simulink model "Simulatore_COMAU_UBHAND_IV_manipolazione_interna"  (Simulink 7.6)
settings: Solver=ode45  StartTime=0.0  StopTime=inf  RelTol=1e-3
values from neighbouring files (symbols this model uses):
  a_f = zeros(4,5)    [KinematicData.m]
  alpha_f = zeros(4,5)    [KinematicData.m]
  d_f = zeros(4,5)    [KinematicData.m]
  dc4_1 = 660    [KinematicData.m]
  T6HAND = [1 0 0 0;0 1 0 0; 0 0 1 357.48+95; 0 0 0 1]    [KinematicData.m]
  teta_d = atan(647.07/130)    [KinematicData.m]
  teta_f = zeros(4,5)    [KinematicData.m]
undefined symbols (not defined in the file or in its folder): a_c, a_w, alpha_c, alpha_w, d_c, d_w, Dt_fingers, teta_c
libraries: simulink x281, dspmtrx3 x171, aerolibutil x163, aerolibconvert2 x64, simulink_extras x46, vrlib x26, aerolibtransform2 x7, dspnetwork x1
(part 7 of 15)

== system Simulatore_COMAU_UBHAND_IV_manipolazione_interna/SCOPES/Direct_kinematic2/Direct_kinematic_hand/Direct_kinematic_index/Subsystem ==
  q : Inport
  alpha : Inport  Port=2
  a : Inport  Port=3
  A_01 : aerolibutil/Create 3x3 Matrix
  Constant : Constant  Value=0
  Constant1 : Constant  Value=[0 0 0 1]  VectorParams1D=off
  Constant5 : Constant  Value=0
  Display : Display
  Gain : Gain  Gain=-1
  Gain1 : Gain  Gain=-1
  Matrix Concatenation : Concatenate  Mode=Multidimensional array  ConcatenateDimension=2
  Matrix Concatenation1 : Concatenate  Mode=Multidimensional array
  Product{,1..5} (6 alike) : Product  RndMeth=Zero
  Trigonometric Function{,1,6..8,11..12} (7 alike) : Trigonometry  Operator=cos
  Trigonometric Function{2..5,9..10,13} (7 alike) : Trigonometry
  Vector Concatenate : Concatenate  NumInputs=3  Mode=Multidimensional array
  Out1 : Outport
  wires:
  Matrix Concatenation1/1 -> Display/1, Out1/1
  Constant1/1 -> Matrix Concatenation1/2
  Matrix Concatenation/1 -> Matrix Concatenation1/1
  Vector Concatenate/1 -> Matrix Concatenation/2
  A_01/1 -> Matrix Concatenation/1
  Constant5/1 -> Vector Concatenate/3
  Product5/1 -> Vector Concatenate/2
  Product4/1 -> Vector Concatenate/1
  Trigonometric Function13/1 -> Product5/2
  Trigonometric Function12/1 -> Product4/2
  a/1 -> Product5/1, Product4/1
  Trigonometric Function11/1 -> A_01/9
  Trigonometric Function10/1 -> A_01/8
  Constant/1 -> A_01/7
  Gain1/1 -> A_01/6
  Product2/1 -> A_01/5
  Trigonometric Function5/1 -> A_01/4
  Product1/1 -> A_01/3
  Gain/1 -> A_01/2
  Trigonometric Function/1 -> A_01/1
  Product/1 -> Gain/1
  Product3/1 -> Gain1/1
  Trigonometric Function9/1 -> Product3/2
  Trigonometric Function8/1 -> Product3/1
  Trigonometric Function7/1 -> Product2/2
  Trigonometric Function6/1 -> Product2/1
  Trigonometric Function4/1 -> Product1/2
  Trigonometric Function3/1 -> Product1/1
  alpha/1 -> Trigonometric Function11/1, Trigonometric Function10/1, Trigonometric Function9/1, Trigonometric Function7/1, Trigonometric Function4/1, Trigonometric Function1/1
  q/1 -> Trigonometric Function13/1, Trigonometric Function12/1, Trigonometric Function8/1, Trigonometric Function6/1, Trigonometric Function5/1, Trigonometric Function3/1, Trigonometric Function2/1, Trigonometric Function/1
  Trigonometric Function1/1 -> Product/2
  Trigonometric Function2/1 -> Product/1

== system Simulatore_COMAU_UBHAND_IV_manipolazione_interna/SCOPES/Direct_kinematic2/Direct_kinematic_hand/Direct_kinematic_index/Subsystem1 ==
  q : Inport
  alpha : Inport  Port=2
  a : Inport  Port=3
  A_01 : aerolibutil/Create 3x3 Matrix
  Constant : Constant  Value=0
  Constant1 : Constant  Value=[0 0 0 1]  VectorParams1D=off
  Constant5 : Constant  Value=0
  Display : Display
  Gain : Gain  Gain=-1
  Gain1 : Gain  Gain=-1
  Matrix Concatenation : Concatenate  Mode=Multidimensional array  ConcatenateDimension=2
  Matrix Concatenation1 : Concatenate  Mode=Multidimensional array
  Product{,1..5} (6 alike) : Product  RndMeth=Zero
  Trigonometric Function{,1,6..8,11..12} (7 alike) : Trigonometry  Operator=cos
  Trigonometric Function{2..5,9..10,13} (7 alike) : Trigonometry
  Vector Concatenate : Concatenate  NumInputs=3  Mode=Multidimensional array
  Out1 : Outport
  wires:
  Trigonometric Function2/1 -> Product/1
  Trigonometric Function1/1 -> Product/2
  q/1 -> Trigonometric Function/1, Trigonometric Function2/1, Trigonometric Function3/1, Trigonometric Function5/1, Trigonometric Function6/1, Trigonometric Function8/1, Trigonometric Function12/1, Trigonometric Function13/1
  alpha/1 -> Trigonometric Function1/1, Trigonometric Function4/1, Trigonometric Function7/1, Trigonometric Function9/1, Trigonometric Function10/1, Trigonometric Function11/1
  Trigonometric Function3/1 -> Product1/1
  Trigonometric Function4/1 -> Product1/2
  Trigonometric Function6/1 -> Product2/1
  Trigonometric Function7/1 -> Product2/2
  Trigonometric Function8/1 -> Product3/1
  Trigonometric Function9/1 -> Product3/2
  Product3/1 -> Gain1/1
  Product/1 -> Gain/1
  Trigonometric Function/1 -> A_01/1
  Gain/1 -> A_01/2
  Product1/1 -> A_01/3
  Trigonometric Function5/1 -> A_01/4
  Product2/1 -> A_01/5
  Gain1/1 -> A_01/6
  Constant/1 -> A_01/7
  Trigonometric Function10/1 -> A_01/8
  Trigonometric Function11/1 -> A_01/9
  a/1 -> Product5/1, Product4/1
  Trigonometric Function12/1 -> Product4/2
  Trigonometric Function13/1 -> Product5/2
  Product4/1 -> Vector Concatenate/1
  Product5/1 -> Vector Concatenate/2
  Constant5/1 -> Vector Concatenate/3
  A_01/1 -> Matrix Concatenation/1
  Vector Concatenate/1 -> Matrix Concatenation/2
  Matrix Concatenation/1 -> Matrix Concatenation1/1
  Constant1/1 -> Matrix Concatenation1/2
  Matrix Concatenation1/1 -> Out1/1, Display/1

== system Simulatore_COMAU_UBHAND_IV_manipolazione_interna/SCOPES/Direct_kinematic2/Direct_kinematic_hand/Direct_kinematic_index/Subsystem2 ==
  q : Inport
  alpha : Inport  Port=2
  a : Inport  Port=3
  A_01 : aerolibutil/Create 3x3 Matrix
  Constant : Constant  Value=0
  Constant1 : Constant  Value=[0 0 0 1]  VectorParams1D=off
  Constant5 : Constant  Value=0
  Display : Display
  Gain : Gain  Gain=-1
  Gain1 : Gain  Gain=-1
  Matrix Concatenation : Concatenate  Mode=Multidimensional array  ConcatenateDimension=2
  Matrix Concatenation1 : Concatenate  Mode=Multidimensional array
  Product{,1..5} (6 alike) : Product  RndMeth=Zero
  Trigonometric Function{,1,6..8,11..12} (7 alike) : Trigonometry  Operator=cos
  Trigonometric Function{2..5,9..10,13} (7 alike) : Trigonometry
  Vector Concatenate : Concatenate  NumInputs=3  Mode=Multidimensional array
  Out1 : Outport
  wires:
  Matrix Concatenation1/1 -> Display/1, Out1/1
  Constant1/1 -> Matrix Concatenation1/2
  Matrix Concatenation/1 -> Matrix Concatenation1/1
  Vector Concatenate/1 -> Matrix Concatenation/2
  A_01/1 -> Matrix Concatenation/1
  Constant5/1 -> Vector Concatenate/3
  Product5/1 -> Vector Concatenate/2
  Product4/1 -> Vector Concatenate/1
  Trigonometric Function13/1 -> Product5/2
  Trigonometric Function12/1 -> Product4/2
  a/1 -> Product5/1, Product4/1
  Trigonometric Function11/1 -> A_01/9
  Trigonometric Function10/1 -> A_01/8
  Constant/1 -> A_01/7
  Gain1/1 -> A_01/6
  Product2/1 -> A_01/5
  Trigonometric Function5/1 -> A_01/4
  Product1/1 -> A_01/3
  Gain/1 -> A_01/2
  Trigonometric Function/1 -> A_01/1
  Product/1 -> Gain/1
  Product3/1 -> Gain1/1
  Trigonometric Function9/1 -> Product3/2
  Trigonometric Function8/1 -> Product3/1
  Trigonometric Function7/1 -> Product2/2
  Trigonometric Function6/1 -> Product2/1
  Trigonometric Function4/1 -> Product1/2
  Trigonometric Function3/1 -> Product1/1
  alpha/1 -> Trigonometric Function11/1, Trigonometric Function10/1, Trigonometric Function9/1, Trigonometric Function7/1, Trigonometric Function4/1, Trigonometric Function1/1
  q/1 -> Trigonometric Function13/1, Trigonometric Function12/1, Trigonometric Function8/1, Trigonometric Function6/1, Trigonometric Function5/1, Trigonometric Function3/1, Trigonometric Function2/1, Trigonometric Function/1
  Trigonometric Function1/1 -> Product/2
  Trigonometric Function2/1 -> Product/1

== system Simulatore_COMAU_UBHAND_IV_manipolazione_interna/SCOPES/Direct_kinematic2/Direct_kinematic_hand/Direct_kinematic_index/Subsystem3 ==
  q : Inport
  alpha : Inport  Port=2
  a : Inport  Port=3
  A_01 : aerolibutil/Create 3x3 Matrix
  Constant : Constant  Value=0
  Constant1 : Constant  Value=[0 0 0 1]  VectorParams1D=off
  Constant5 : Constant  Value=0
  Display : Display
  Gain : Gain  Gain=-1
  Gain1 : Gain  Gain=-1
  Matrix Concatenation : Concatenate  Mode=Multidimensional array  ConcatenateDimension=2
  Matrix Concatenation1 : Concatenate  Mode=Multidimensional array
  Product{,1..5} (6 alike) : Product  RndMeth=Zero
  Trigonometric Function{,1,6..8,11..12} (7 alike) : Trigonometry  Operator=cos
  Trigonometric Function{2..5,9..10,13} (7 alike) : Trigonometry
  Vector Concatenate : Concatenate  NumInputs=3  Mode=Multidimensional array
  Out1 : Outport
  wires:
  Trigonometric Function2/1 -> Product/1
  Trigonometric Function1/1 -> Product/2
  q/1 -> Trigonometric Function/1, Trigonometric Function2/1, Trigonometric Function3/1, Trigonometric Function5/1, Trigonometric Function6/1, Trigonometric Function8/1, Trigonometric Function12/1, Trigonometric Function13/1
  alpha/1 -> Trigonometric Function1/1, Trigonometric Function4/1, Trigonometric Function7/1, Trigonometric Function9/1, Trigonometric Function10/1, Trigonometric Function11/1
  Trigonometric Function3/1 -> Product1/1
  Trigonometric Function4/1 -> Product1/2
  Trigonometric Function6/1 -> Product2/1
  Trigonometric Function7/1 -> Product2/2
  Trigonometric Function8/1 -> Product3/1
  Trigonometric Function9/1 -> Product3/2
  Product3/1 -> Gain1/1
  Product/1 -> Gain/1
  Trigonometric Function/1 -> A_01/1
  Gain/1 -> A_01/2
  Product1/1 -> A_01/3
  Trigonometric Function5/1 -> A_01/4
  Product2/1 -> A_01/5
  Gain1/1 -> A_01/6
  Constant/1 -> A_01/7
  Trigonometric Function10/1 -> A_01/8
  Trigonometric Function11/1 -> A_01/9
  a/1 -> Product5/1, Product4/1
  Trigonometric Function12/1 -> Product4/2
  Trigonometric Function13/1 -> Product5/2
  Product4/1 -> Vector Concatenate/1
  Product5/1 -> Vector Concatenate/2
  Constant5/1 -> Vector Concatenate/3
  A_01/1 -> Matrix Concatenation/1
  Vector Concatenate/1 -> Matrix Concatenation/2
  Matrix Concatenation/1 -> Matrix Concatenation1/1
  Constant1/1 -> Matrix Concatenation1/2
  Matrix Concatenation1/1 -> Out1/1, Display/1

== system Simulatore_COMAU_UBHAND_IV_manipolazione_interna/SCOPES/Direct_kinematic2/Direct_kinematic_hand/Direct_kinematic_little/Subsystem ==
  q : Inport
  alpha : Inport  Port=2
  a : Inport  Port=3
  A_01 : aerolibutil/Create 3x3 Matrix
  Constant : Constant  Value=0
  Constant1 : Constant  Value=[0 0 0 1]  VectorParams1D=off
  Constant5 : Constant  Value=0
  Display : Display
  Gain : Gain  Gain=-1
  Gain1 : Gain  Gain=-1
  Matrix Concatenation : Concatenate  Mode=Multidimensional array  ConcatenateDimension=2
  Matrix Concatenation1 : Concatenate  Mode=Multidimensional array
  Product{,1..5} (6 alike) : Product  RndMeth=Zero
  Trigonometric Function{,1,6..8,11..12} (7 alike) : Trigonometry  Operator=cos
  Trigonometric Function{2..5,9..10,13} (7 alike) : Trigonometry
  Vector Concatenate : Concatenate  NumInputs=3  Mode=Multidimensional array
  Out1 : Outport
  wires:
  Matrix Concatenation1/1 -> Display/1, Out1/1
  Constant1/1 -> Matrix Concatenation1/2
  Matrix Concatenation/1 -> Matrix Concatenation1/1
  Vector Concatenate/1 -> Matrix Concatenation/2
  A_01/1 -> Matrix Concatenation/1
  Constant5/1 -> Vector Concatenate/3
  Product5/1 -> Vector Concatenate/2
  Product4/1 -> Vector Concatenate/1
  Trigonometric Function13/1 -> Product5/2
  Trigonometric Function12/1 -> Product4/2
  a/1 -> Product5/1, Product4/1
  Trigonometric Function11/1 -> A_01/9
  Trigonometric Function10/1 -> A_01/8
  Constant/1 -> A_01/7
  Gain1/1 -> A_01/6
  Product2/1 -> A_01/5
  Trigonometric Function5/1 -> A_01/4
  Product1/1 -> A_01/3
  Gain/1 -> A_01/2
  Trigonometric Function/1 -> A_01/1
  Product/1 -> Gain/1
  Product3/1 -> Gain1/1
  Trigonometric Function9/1 -> Product3/2
  Trigonometric Function8/1 -> Product3/1
  Trigonometric Function7/1 -> Product2/2
  Trigonometric Function6/1 -> Product2/1
  Trigonometric Function4/1 -> Product1/2
  Trigonometric Function3/1 -> Product1/1
  alpha/1 -> Trigonometric Function11/1, Trigonometric Function10/1, Trigonometric Function9/1, Trigonometric Function7/1, Trigonometric Function4/1, Trigonometric Function1/1
  q/1 -> Trigonometric Function13/1, Trigonometric Function12/1, Trigonometric Function8/1, Trigonometric Function6/1, Trigonometric Function5/1, Trigonometric Function3/1, Trigonometric Function2/1, Trigonometric Function/1
  Trigonometric Function1/1 -> Product/2
  Trigonometric Function2/1 -> Product/1

== system Simulatore_COMAU_UBHAND_IV_manipolazione_interna/SCOPES/Direct_kinematic2/Direct_kinematic_hand/Direct_kinematic_little/Subsystem1 ==
  q : Inport
  alpha : Inport  Port=2
  a : Inport  Port=3
  A_01 : aerolibutil/Create 3x3 Matrix
  Constant : Constant  Value=0
  Constant1 : Constant  Value=[0 0 0 1]  VectorParams1D=off
  Constant5 : Constant  Value=0
  Display : Display
  Gain : Gain  Gain=-1
  Gain1 : Gain  Gain=-1
  Matrix Concatenation : Concatenate  Mode=Multidimensional array  ConcatenateDimension=2
  Matrix Concatenation1 : Concatenate  Mode=Multidimensional array
  Product{,1..5} (6 alike) : Product  RndMeth=Zero
  Trigonometric Function{,1,6..8,11..12} (7 alike) : Trigonometry  Operator=cos
  Trigonometric Function{2..5,9..10,13} (7 alike) : Trigonometry
  Vector Concatenate : Concatenate  NumInputs=3  Mode=Multidimensional array
  Out1 : Outport
  wires:
  Trigonometric Function2/1 -> Product/1
  Trigonometric Function1/1 -> Product/2
  q/1 -> Trigonometric Function/1, Trigonometric Function2/1, Trigonometric Function3/1, Trigonometric Function5/1, Trigonometric Function6/1, Trigonometric Function8/1, Trigonometric Function12/1, Trigonometric Function13/1
  alpha/1 -> Trigonometric Function1/1, Trigonometric Function4/1, Trigonometric Function7/1, Trigonometric Function9/1, Trigonometric Function10/1, Trigonometric Function11/1
  Trigonometric Function3/1 -> Product1/1
  Trigonometric Function4/1 -> Product1/2
  Trigonometric Function6/1 -> Product2/1
  Trigonometric Function7/1 -> Product2/2
  Trigonometric Function8/1 -> Product3/1
  Trigonometric Function9/1 -> Product3/2
  Product3/1 -> Gain1/1
  Product/1 -> Gain/1
  Trigonometric Function/1 -> A_01/1
  Gain/1 -> A_01/2
  Product1/1 -> A_01/3
  Trigonometric Function5/1 -> A_01/4
  Product2/1 -> A_01/5
  Gain1/1 -> A_01/6
  Constant/1 -> A_01/7
  Trigonometric Function10/1 -> A_01/8
  Trigonometric Function11/1 -> A_01/9
  a/1 -> Product5/1, Product4/1
  Trigonometric Function12/1 -> Product4/2
  Trigonometric Function13/1 -> Product5/2
  Product4/1 -> Vector Concatenate/1
  Product5/1 -> Vector Concatenate/2
  Constant5/1 -> Vector Concatenate/3
  A_01/1 -> Matrix Concatenation/1
  Vector Concatenate/1 -> Matrix Concatenation/2
  Matrix Concatenation/1 -> Matrix Concatenation1/1
  Constant1/1 -> Matrix Concatenation1/2
  Matrix Concatenation1/1 -> Out1/1, Display/1

== system Simulatore_COMAU_UBHAND_IV_manipolazione_interna/SCOPES/Direct_kinematic2/Direct_kinematic_hand/Direct_kinematic_little/Subsystem2 ==
  q : Inport
  alpha : Inport  Port=2
  a : Inport  Port=3
  A_01 : aerolibutil/Create 3x3 Matrix
  Constant : Constant  Value=0
  Constant1 : Constant  Value=[0 0 0 1]  VectorParams1D=off
  Constant5 : Constant  Value=0
  Display : Display
  Gain : Gain  Gain=-1
  Gain1 : Gain  Gain=-1
  Matrix Concatenation : Concatenate  Mode=Multidimensional array  ConcatenateDimension=2
  Matrix Concatenation1 : Concatenate  Mode=Multidimensional array
  Product{,1..5} (6 alike) : Product  RndMeth=Zero
  Trigonometric Function{,1,6..8,11..12} (7 alike) : Trigonometry  Operator=cos
  Trigonometric Function{2..5,9..10,13} (7 alike) : Trigonometry
  Vector Concatenate : Concatenate  NumInputs=3  Mode=Multidimensional array
  Out1 : Outport
  wires:
  Matrix Concatenation1/1 -> Display/1, Out1/1
  Constant1/1 -> Matrix Concatenation1/2
  Matrix Concatenation/1 -> Matrix Concatenation1/1
  Vector Concatenate/1 -> Matrix Concatenation/2
  A_01/1 -> Matrix Concatenation/1
  Constant5/1 -> Vector Concatenate/3
  Product5/1 -> Vector Concatenate/2
  Product4/1 -> Vector Concatenate/1
  Trigonometric Function13/1 -> Product5/2
  Trigonometric Function12/1 -> Product4/2
  a/1 -> Product5/1, Product4/1
  Trigonometric Function11/1 -> A_01/9
  Trigonometric Function10/1 -> A_01/8
  Constant/1 -> A_01/7
  Gain1/1 -> A_01/6
  Product2/1 -> A_01/5
  Trigonometric Function5/1 -> A_01/4
  Product1/1 -> A_01/3
  Gain/1 -> A_01/2
  Trigonometric Function/1 -> A_01/1
  Product/1 -> Gain/1
  Product3/1 -> Gain1/1
  Trigonometric Function9/1 -> Product3/2
  Trigonometric Function8/1 -> Product3/1
  Trigonometric Function7/1 -> Product2/2
  Trigonometric Function6/1 -> Product2/1
  Trigonometric Function4/1 -> Product1/2
  Trigonometric Function3/1 -> Product1/1
  alpha/1 -> Trigonometric Function11/1, Trigonometric Function10/1, Trigonometric Function9/1, Trigonometric Function7/1, Trigonometric Function4/1, Trigonometric Function1/1
  q/1 -> Trigonometric Function13/1, Trigonometric Function12/1, Trigonometric Function8/1, Trigonometric Function6/1, Trigonometric Function5/1, Trigonometric Function3/1, Trigonometric Function2/1, Trigonometric Function/1
  Trigonometric Function1/1 -> Product/2
  Trigonometric Function2/1 -> Product/1

== system Simulatore_COMAU_UBHAND_IV_manipolazione_interna/SCOPES/Direct_kinematic2/Direct_kinematic_hand/Direct_kinematic_little/Subsystem3 ==
  q : Inport
  alpha : Inport  Port=2
  a : Inport  Port=3
  A_01 : aerolibutil/Create 3x3 Matrix
  Constant : Constant  Value=0
  Constant1 : Constant  Value=[0 0 0 1]  VectorParams1D=off
  Constant5 : Constant  Value=0
  Display : Display
  Gain : Gain  Gain=-1
  Gain1 : Gain  Gain=-1
  Matrix Concatenation : Concatenate  Mode=Multidimensional array  ConcatenateDimension=2
  Matrix Concatenation1 : Concatenate  Mode=Multidimensional array
  Product{,1..5} (6 alike) : Product  RndMeth=Zero
  Trigonometric Function{,1,6..8,11..12} (7 alike) : Trigonometry  Operator=cos
  Trigonometric Function{2..5,9..10,13} (7 alike) : Trigonometry
  Vector Concatenate : Concatenate  NumInputs=3  Mode=Multidimensional array
  Out1 : Outport
  wires:
  Trigonometric Function2/1 -> Product/1
  Trigonometric Function1/1 -> Product/2
  q/1 -> Trigonometric Function/1, Trigonometric Function2/1, Trigonometric Function3/1, Trigonometric Function5/1, Trigonometric Function6/1, Trigonometric Function8/1, Trigonometric Function12/1, Trigonometric Function13/1
  alpha/1 -> Trigonometric Function1/1, Trigonometric Function4/1, Trigonometric Function7/1, Trigonometric Function9/1, Trigonometric Function10/1, Trigonometric Function11/1
  Trigonometric Function3/1 -> Product1/1
  Trigonometric Function4/1 -> Product1/2
  Trigonometric Function6/1 -> Product2/1
  Trigonometric Function7/1 -> Product2/2
  Trigonometric Function8/1 -> Product3/1
  Trigonometric Function9/1 -> Product3/2
  Product3/1 -> Gain1/1
  Product/1 -> Gain/1
  Trigonometric Function/1 -> A_01/1
  Gain/1 -> A_01/2
  Product1/1 -> A_01/3
  Trigonometric Function5/1 -> A_01/4
  Product2/1 -> A_01/5
  Gain1/1 -> A_01/6
  Constant/1 -> A_01/7
  Trigonometric Function10/1 -> A_01/8
  Trigonometric Function11/1 -> A_01/9
  a/1 -> Product5/1, Product4/1
  Trigonometric Function12/1 -> Product4/2
  Trigonometric Function13/1 -> Product5/2
  Product4/1 -> Vector Concatenate/1
  Product5/1 -> Vector Concatenate/2
  Constant5/1 -> Vector Concatenate/3
  A_01/1 -> Matrix Concatenation/1
  Vector Concatenate/1 -> Matrix Concatenation/2
  Matrix Concatenation/1 -> Matrix Concatenation1/1
  Constant1/1 -> Matrix Concatenation1/2
  Matrix Concatenation1/1 -> Out1/1, Display/1

== system Simulatore_COMAU_UBHAND_IV_manipolazione_interna/SCOPES/Direct_kinematic2/Direct_kinematic_hand/Direct_kinematic_middle/Subsystem ==
  q : Inport
  alpha : Inport  Port=2
  a : Inport  Port=3
  A_01 : aerolibutil/Create 3x3 Matrix
  Constant : Constant  Value=0
  Constant1 : Constant  Value=[0 0 0 1]  VectorParams1D=off
  Constant5 : Constant  Value=0
  Display : Display
  Gain : Gain  Gain=-1
  Gain1 : Gain  Gain=-1
  Matrix Concatenation : Concatenate  Mode=Multidimensional array  ConcatenateDimension=2
  Matrix Concatenation1 : Concatenate  Mode=Multidimensional array
  Product{,1..5} (6 alike) : Product  RndMeth=Zero
  Trigonometric Function{,1,6..8,11..12} (7 alike) : Trigonometry  Operator=cos
  Trigonometric Function{2..5,9..10,13} (7 alike) : Trigonometry
  Vector Concatenate : Concatenate  NumInputs=3  Mode=Multidimensional array
  Out1 : Outport
  wires:
  Trigonometric Function2/1 -> Product/1
  Trigonometric Function1/1 -> Product/2
  q/1 -> Trigonometric Function/1, Trigonometric Function2/1, Trigonometric Function3/1, Trigonometric Function5/1, Trigonometric Function6/1, Trigonometric Function8/1, Trigonometric Function12/1, Trigonometric Function13/1
  alpha/1 -> Trigonometric Function1/1, Trigonometric Function4/1, Trigonometric Function7/1, Trigonometric Function9/1, Trigonometric Function10/1, Trigonometric Function11/1
  Trigonometric Function3/1 -> Product1/1
  Trigonometric Function4/1 -> Product1/2
  Trigonometric Function6/1 -> Product2/1
  Trigonometric Function7/1 -> Product2/2
  Trigonometric Function8/1 -> Product3/1
  Trigonometric Function9/1 -> Product3/2
  Product3/1 -> Gain1/1
  Product/1 -> Gain/1
  Trigonometric Function/1 -> A_01/1
  Gain/1 -> A_01/2
  Product1/1 -> A_01/3
  Trigonometric Function5/1 -> A_01/4
  Product2/1 -> A_01/5
  Gain1/1 -> A_01/6
  Constant/1 -> A_01/7
  Trigonometric Function10/1 -> A_01/8
  Trigonometric Function11/1 -> A_01/9
  a/1 -> Product4/1, Product5/1
  Trigonometric Function12/1 -> Product4/2
  Trigonometric Function13/1 -> Product5/2
  Product4/1 -> Vector Concatenate/1
  Product5/1 -> Vector Concatenate/2
  Constant5/1 -> Vector Concatenate/3
  A_01/1 -> Matrix Concatenation/1
  Vector Concatenate/1 -> Matrix Concatenation/2
  Matrix Concatenation/1 -> Matrix Concatenation1/1
  Constant1/1 -> Matrix Concatenation1/2
  Matrix Concatenation1/1 -> Out1/1, Display/1

== system Simulatore_COMAU_UBHAND_IV_manipolazione_interna/SCOPES/Direct_kinematic2/Direct_kinematic_hand/Direct_kinematic_middle/Subsystem1 ==
  q : Inport
  alpha : Inport  Port=2
  a : Inport  Port=3
  A_01 : aerolibutil/Create 3x3 Matrix
  Constant : Constant  Value=0
  Constant1 : Constant  Value=[0 0 0 1]  VectorParams1D=off
  Constant5 : Constant  Value=0
  Display : Display
  Gain : Gain  Gain=-1
  Gain1 : Gain  Gain=-1
  Matrix Concatenation : Concatenate  Mode=Multidimensional array  ConcatenateDimension=2
  Matrix Concatenation1 : Concatenate  Mode=Multidimensional array
  Product{,1..5} (6 alike) : Product  RndMeth=Zero
  Trigonometric Function{,1,6..8,11..12} (7 alike) : Trigonometry  Operator=cos
  Trigonometric Function{2..5,9..10,13} (7 alike) : Trigonometry
  Vector Concatenate : Concatenate  NumInputs=3  Mode=Multidimensional array
  Out1 : Outport
  wires:
  Matrix Concatenation1/1 -> Display/1, Out1/1
  Constant1/1 -> Matrix Concatenation1/2
  Matrix Concatenation/1 -> Matrix Concatenation1/1
  Vector Concatenate/1 -> Matrix Concatenation/2
  A_01/1 -> Matrix Concatenation/1
  Constant5/1 -> Vector Concatenate/3
  Product5/1 -> Vector Concatenate/2
  Product4/1 -> Vector Concatenate/1
  Trigonometric Function13/1 -> Product5/2
  Trigonometric Function12/1 -> Product4/2
  a/1 -> Product4/1, Product5/1
  Trigonometric Function11/1 -> A_01/9
  Trigonometric Function10/1 -> A_01/8
  Constant/1 -> A_01/7
  Gain1/1 -> A_01/6
  Product2/1 -> A_01/5
  Trigonometric Function5/1 -> A_01/4
  Product1/1 -> A_01/3
  Gain/1 -> A_01/2
  Trigonometric Function/1 -> A_01/1
  Product/1 -> Gain/1
  Product3/1 -> Gain1/1
  Trigonometric Function9/1 -> Product3/2
  Trigonometric Function8/1 -> Product3/1
  Trigonometric Function7/1 -> Product2/2
  Trigonometric Function6/1 -> Product2/1
  Trigonometric Function4/1 -> Product1/2
  Trigonometric Function3/1 -> Product1/1
  alpha/1 -> Trigonometric Function11/1, Trigonometric Function10/1, Trigonometric Function9/1, Trigonometric Function7/1, Trigonometric Function4/1, Trigonometric Function1/1
  q/1 -> Trigonometric Function13/1, Trigonometric Function12/1, Trigonometric Function8/1, Trigonometric Function6/1, Trigonometric Function5/1, Trigonometric Function3/1, Trigonometric Function2/1, Trigonometric Function/1
  Trigonometric Function1/1 -> Product/2
  Trigonometric Function2/1 -> Product/1

== system Simulatore_COMAU_UBHAND_IV_manipolazione_interna/SCOPES/Direct_kinematic2/Direct_kinematic_hand/Direct_kinematic_middle/Subsystem2 ==
  q : Inport
  alpha : Inport  Port=2
  a : Inport  Port=3
  A_01 : aerolibutil/Create 3x3 Matrix
  Constant : Constant  Value=0
  Constant1 : Constant  Value=[0 0 0 1]  VectorParams1D=off
  Constant5 : Constant  Value=0
  Display : Display
  Gain : Gain  Gain=-1
  Gain1 : Gain  Gain=-1
  Matrix Concatenation : Concatenate  Mode=Multidimensional array  ConcatenateDimension=2
  Matrix Concatenation1 : Concatenate  Mode=Multidimensional array
  Product{,1..5} (6 alike) : Product  RndMeth=Zero
  Trigonometric Function{,1,6..8,11..12} (7 alike) : Trigonometry  Operator=cos
  Trigonometric Function{2..5,9..10,13} (7 alike) : Trigonometry
  Vector Concatenate : Concatenate  NumInputs=3  Mode=Multidimensional array
  Out1 : Outport
  wires:
  Trigonometric Function2/1 -> Product/1
  Trigonometric Function1/1 -> Product/2
  q/1 -> Trigonometric Function/1, Trigonometric Function2/1, Trigonometric Function3/1, Trigonometric Function5/1, Trigonometric Function6/1, Trigonometric Function8/1, Trigonometric Function12/1, Trigonometric Function13/1
  alpha/1 -> Trigonometric Function1/1, Trigonometric Function4/1, Trigonometric Function7/1, Trigonometric Function9/1, Trigonometric Function10/1, Trigonometric Function11/1
  Trigonometric Function3/1 -> Product1/1
  Trigonometric Function4/1 -> Product1/2
  Trigonometric Function6/1 -> Product2/1
  Trigonometric Function7/1 -> Product2/2
  Trigonometric Function8/1 -> Product3/1
  Trigonometric Function9/1 -> Product3/2
  Product3/1 -> Gain1/1
  Product/1 -> Gain/1
  Trigonometric Function/1 -> A_01/1
  Gain/1 -> A_01/2
  Product1/1 -> A_01/3
  Trigonometric Function5/1 -> A_01/4
  Product2/1 -> A_01/5
  Gain1/1 -> A_01/6
  Constant/1 -> A_01/7
  Trigonometric Function10/1 -> A_01/8
  Trigonometric Function11/1 -> A_01/9
  a/1 -> Product4/1, Product5/1
  Trigonometric Function12/1 -> Product4/2
  Trigonometric Function13/1 -> Product5/2
  Product4/1 -> Vector Concatenate/1
  Product5/1 -> Vector Concatenate/2
  Constant5/1 -> Vector Concatenate/3
  A_01/1 -> Matrix Concatenation/1
  Vector Concatenate/1 -> Matrix Concatenation/2
  Matrix Concatenation/1 -> Matrix Concatenation1/1
  Constant1/1 -> Matrix Concatenation1/2
  Matrix Concatenation1/1 -> Out1/1, Display/1

== system Simulatore_COMAU_UBHAND_IV_manipolazione_interna/SCOPES/Direct_kinematic2/Direct_kinematic_hand/Direct_kinematic_middle/Subsystem3 ==
  q : Inport
  alpha : Inport  Port=2
  a : Inport  Port=3
  A_01 : aerolibutil/Create 3x3 Matrix
  Constant : Constant  Value=0
  Constant1 : Constant  Value=[0 0 0 1]  VectorParams1D=off
  Constant5 : Constant  Value=0
  Display : Display
  Gain : Gain  Gain=-1
  Gain1 : Gain  Gain=-1
  Matrix Concatenation : Concatenate  Mode=Multidimensional array  ConcatenateDimension=2
  Matrix Concatenation1 : Concatenate  Mode=Multidimensional array
  Product{,1..5} (6 alike) : Product  RndMeth=Zero
  Trigonometric Function{,1,6..8,11..12} (7 alike) : Trigonometry  Operator=cos
  Trigonometric Function{2..5,9..10,13} (7 alike) : Trigonometry
  Vector Concatenate : Concatenate  NumInputs=3  Mode=Multidimensional array
  Out1 : Outport
  wires:
  Matrix Concatenation1/1 -> Display/1, Out1/1
  Constant1/1 -> Matrix Concatenation1/2
  Matrix Concatenation/1 -> Matrix Concatenation1/1
  Vector Concatenate/1 -> Matrix Concatenation/2
  A_01/1 -> Matrix Concatenation/1
  Constant5/1 -> Vector Concatenate/3
  Product5/1 -> Vector Concatenate/2
  Product4/1 -> Vector Concatenate/1
  Trigonometric Function13/1 -> Product5/2
  Trigonometric Function12/1 -> Product4/2
  a/1 -> Product4/1, Product5/1
  Trigonometric Function11/1 -> A_01/9
  Trigonometric Function10/1 -> A_01/8
  Constant/1 -> A_01/7
  Gain1/1 -> A_01/6
  Product2/1 -> A_01/5
  Trigonometric Function5/1 -> A_01/4
  Product1/1 -> A_01/3
  Gain/1 -> A_01/2
  Trigonometric Function/1 -> A_01/1
  Product/1 -> Gain/1
  Product3/1 -> Gain1/1
  Trigonometric Function9/1 -> Product3/2
  Trigonometric Function8/1 -> Product3/1
  Trigonometric Function7/1 -> Product2/2
  Trigonometric Function6/1 -> Product2/1
  Trigonometric Function4/1 -> Product1/2
  Trigonometric Function3/1 -> Product1/1
  alpha/1 -> Trigonometric Function11/1, Trigonometric Function10/1, Trigonometric Function9/1, Trigonometric Function7/1, Trigonometric Function4/1, Trigonometric Function1/1
  q/1 -> Trigonometric Function13/1, Trigonometric Function12/1, Trigonometric Function8/1, Trigonometric Function6/1, Trigonometric Function5/1, Trigonometric Function3/1, Trigonometric Function2/1, Trigonometric Function/1
  Trigonometric Function1/1 -> Product/2
  Trigonometric Function2/1 -> Product/1

== system Simulatore_COMAU_UBHAND_IV_manipolazione_interna/SCOPES/Direct_kinematic2/Direct_kinematic_hand/Direct_kinematic_ring/Subsystem ==
  q : Inport
  alpha : Inport  Port=2
  a : Inport  Port=3
  A_01 : aerolibutil/Create 3x3 Matrix
  Constant : Constant  Value=0
  Constant1 : Constant  Value=[0 0 0 1]  VectorParams1D=off
  Constant5 : Constant  Value=0
  Display : Display
  Gain : Gain  Gain=-1
  Gain1 : Gain  Gain=-1
  Matrix Concatenation : Concatenate  Mode=Multidimensional array  ConcatenateDimension=2
  Matrix Concatenation1 : Concatenate  Mode=Multidimensional array
  Product{,1..5} (6 alike) : Product  RndMeth=Zero
  Trigonometric Function{,1,6..8,11..12} (7 alike) : Trigonometry  Operator=cos
  Trigonometric Function{2..5,9..10,13} (7 alike) : Trigonometry
  Vector Concatenate : Concatenate  NumInputs=3  Mode=Multidimensional array
  Out1 : Outport
  wires:
  Matrix Concatenation1/1 -> Display/1, Out1/1
  Constant1/1 -> Matrix Concatenation1/2
  Matrix Concatenation/1 -> Matrix Concatenation1/1
  Vector Concatenate/1 -> Matrix Concatenation/2
  A_01/1 -> Matrix Concatenation/1
  Constant5/1 -> Vector Concatenate/3
  Product5/1 -> Vector Concatenate/2
  Product4/1 -> Vector Concatenate/1
  Trigonometric Function13/1 -> Product5/2
  Trigonometric Function12/1 -> Product4/2
  a/1 -> Product5/1, Product4/1
  Trigonometric Function11/1 -> A_01/9
  Trigonometric Function10/1 -> A_01/8
  Constant/1 -> A_01/7
  Gain1/1 -> A_01/6
  Product2/1 -> A_01/5
  Trigonometric Function5/1 -> A_01/4
  Product1/1 -> A_01/3
  Gain/1 -> A_01/2
  Trigonometric Function/1 -> A_01/1
  Product/1 -> Gain/1
  Product3/1 -> Gain1/1
  Trigonometric Function9/1 -> Product3/2
  Trigonometric Function8/1 -> Product3/1
  Trigonometric Function7/1 -> Product2/2
  Trigonometric Function6/1 -> Product2/1
  Trigonometric Function4/1 -> Product1/2
  Trigonometric Function3/1 -> Product1/1
  alpha/1 -> Trigonometric Function11/1, Trigonometric Function10/1, Trigonometric Function9/1, Trigonometric Function7/1, Trigonometric Function4/1, Trigonometric Function1/1
  q/1 -> Trigonometric Function13/1, Trigonometric Function12/1, Trigonometric Function8/1, Trigonometric Function6/1, Trigonometric Function5/1, Trigonometric Function3/1, Trigonometric Function2/1, Trigonometric Function/1
  Trigonometric Function1/1 -> Product/2
  Trigonometric Function2/1 -> Product/1

== system Simulatore_COMAU_UBHAND_IV_manipolazione_interna/SCOPES/Direct_kinematic2/Direct_kinematic_hand/Direct_kinematic_ring/Subsystem1 ==
  q : Inport
  alpha : Inport  Port=2
  a : Inport  Port=3
  A_01 : aerolibutil/Create 3x3 Matrix
  Constant : Constant  Value=0
  Constant1 : Constant  Value=[0 0 0 1]  VectorParams1D=off
  Constant5 : Constant  Value=0
  Display : Display
  Gain : Gain  Gain=-1
  Gain1 : Gain  Gain=-1
  Matrix Concatenation : Concatenate  Mode=Multidimensional array  ConcatenateDimension=2
  Matrix Concatenation1 : Concatenate  Mode=Multidimensional array
  Product{,1..5} (6 alike) : Product  RndMeth=Zero
  Trigonometric Function{,1,6..8,11..12} (7 alike) : Trigonometry  Operator=cos
  Trigonometric Function{2..5,9..10,13} (7 alike) : Trigonometry
  Vector Concatenate : Concatenate  NumInputs=3  Mode=Multidimensional array
  Out1 : Outport
  wires:
  Trigonometric Function2/1 -> Product/1
  Trigonometric Function1/1 -> Product/2
  q/1 -> Trigonometric Function/1, Trigonometric Function2/1, Trigonometric Function3/1, Trigonometric Function5/1, Trigonometric Function6/1, Trigonometric Function8/1, Trigonometric Function12/1, Trigonometric Function13/1
  alpha/1 -> Trigonometric Function1/1, Trigonometric Function4/1, Trigonometric Function7/1, Trigonometric Function9/1, Trigonometric Function10/1, Trigonometric Function11/1
  Trigonometric Function3/1 -> Product1/1
  Trigonometric Function4/1 -> Product1/2
  Trigonometric Function6/1 -> Product2/1
  Trigonometric Function7/1 -> Product2/2
  Trigonometric Function8/1 -> Product3/1
  Trigonometric Function9/1 -> Product3/2
  Product3/1 -> Gain1/1
  Product/1 -> Gain/1
  Trigonometric Function/1 -> A_01/1
  Gain/1 -> A_01/2
  Product1/1 -> A_01/3
  Trigonometric Function5/1 -> A_01/4
  Product2/1 -> A_01/5
  Gain1/1 -> A_01/6
  Constant/1 -> A_01/7
  Trigonometric Function10/1 -> A_01/8
  Trigonometric Function11/1 -> A_01/9
  a/1 -> Product5/1, Product4/1
  Trigonometric Function12/1 -> Product4/2
  Trigonometric Function13/1 -> Product5/2
  Product4/1 -> Vector Concatenate/1
  Product5/1 -> Vector Concatenate/2
  Constant5/1 -> Vector Concatenate/3
  A_01/1 -> Matrix Concatenation/1
  Vector Concatenate/1 -> Matrix Concatenation/2
  Matrix Concatenation/1 -> Matrix Concatenation1/1
  Constant1/1 -> Matrix Concatenation1/2
  Matrix Concatenation1/1 -> Out1/1, Display/1

== system Simulatore_COMAU_UBHAND_IV_manipolazione_interna/SCOPES/Direct_kinematic2/Direct_kinematic_hand/Direct_kinematic_ring/Subsystem2 ==
  q : Inport
  alpha : Inport  Port=2
  a : Inport  Port=3
  A_01 : aerolibutil/Create 3x3 Matrix
  Constant : Constant  Value=0
  Constant1 : Constant  Value=[0 0 0 1]  VectorParams1D=off
  Constant5 : Constant  Value=0
  Display : Display
  Gain : Gain  Gain=-1
  Gain1 : Gain  Gain=-1
  Matrix Concatenation : Concatenate  Mode=Multidimensional array  ConcatenateDimension=2
  Matrix Concatenation1 : Concatenate  Mode=Multidimensional array
  Product{,1..5} (6 alike) : Product  RndMeth=Zero
  Trigonometric Function{,1,6..8,11..12} (7 alike) : Trigonometry  Operator=cos
  Trigonometric Function{2..5,9..10,13} (7 alike) : Trigonometry
  Vector Concatenate : Concatenate  NumInputs=3  Mode=Multidimensional array
  Out1 : Outport
  wires:
  Matrix Concatenation1/1 -> Display/1, Out1/1
  Constant1/1 -> Matrix Concatenation1/2
  Matrix Concatenation/1 -> Matrix Concatenation1/1
  Vector Concatenate/1 -> Matrix Concatenation/2
  A_01/1 -> Matrix Concatenation/1
  Constant5/1 -> Vector Concatenate/3
  Product5/1 -> Vector Concatenate/2
  Product4/1 -> Vector Concatenate/1
  Trigonometric Function13/1 -> Product5/2
  Trigonometric Function12/1 -> Product4/2
  a/1 -> Product5/1, Product4/1
  Trigonometric Function11/1 -> A_01/9
  Trigonometric Function10/1 -> A_01/8
  Constant/1 -> A_01/7
  Gain1/1 -> A_01/6
  Product2/1 -> A_01/5
  Trigonometric Function5/1 -> A_01/4
  Product1/1 -> A_01/3
  Gain/1 -> A_01/2
  Trigonometric Function/1 -> A_01/1
  Product/1 -> Gain/1
  Product3/1 -> Gain1/1
  Trigonometric Function9/1 -> Product3/2
  Trigonometric Function8/1 -> Product3/1
  Trigonometric Function7/1 -> Product2/2
  Trigonometric Function6/1 -> Product2/1
  Trigonometric Function4/1 -> Product1/2
  Trigonometric Function3/1 -> Product1/1
  alpha/1 -> Trigonometric Function11/1, Trigonometric Function10/1, Trigonometric Function9/1, Trigonometric Function7/1, Trigonometric Function4/1, Trigonometric Function1/1
  q/1 -> Trigonometric Function13/1, Trigonometric Function12/1, Trigonometric Function8/1, Trigonometric Function6/1, Trigonometric Function5/1, Trigonometric Function3/1, Trigonometric Function2/1, Trigonometric Function/1
  Trigonometric Function1/1 -> Product/2
  Trigonometric Function2/1 -> Product/1

== system Simulatore_COMAU_UBHAND_IV_manipolazione_interna/SCOPES/Direct_kinematic2/Direct_kinematic_hand/Direct_kinematic_ring/Subsystem3 ==
  q : Inport
  alpha : Inport  Port=2
  a : Inport  Port=3
  A_01 : aerolibutil/Create 3x3 Matrix
  Constant : Constant  Value=0
  Constant1 : Constant  Value=[0 0 0 1]  VectorParams1D=off
  Constant5 : Constant  Value=0
  Display : Display
  Gain : Gain  Gain=-1
  Gain1 : Gain  Gain=-1
  Matrix Concatenation : Concatenate  Mode=Multidimensional array  ConcatenateDimension=2
  Matrix Concatenation1 : Concatenate  Mode=Multidimensional array
  Product{,1..5} (6 alike) : Product  RndMeth=Zero
  Trigonometric Function{,1,6..8,11..12} (7 alike) : Trigonometry  Operator=cos
  Trigonometric Function{2..5,9..10,13} (7 alike) : Trigonometry
  Vector Concatenate : Concatenate  NumInputs=3  Mode=Multidimensional array
  Out1 : Outport
  wires:
  Trigonometric Function2/1 -> Product/1
  Trigonometric Function1/1 -> Product/2
  q/1 -> Trigonometric Function/1, Trigonometric Function2/1, Trigonometric Function3/1, Trigonometric Function5/1, Trigonometric Function6/1, Trigonometric Function8/1, Trigonometric Function12/1, Trigonometric Function13/1
  alpha/1 -> Trigonometric Function1/1, Trigonometric Function4/1, Trigonometric Function7/1, Trigonometric Function9/1, Trigonometric Function10/1, Trigonometric Function11/1
  Trigonometric Function3/1 -> Product1/1
  Trigonometric Function4/1 -> Product1/2
  Trigonometric Function6/1 -> Product2/1
  Trigonometric Function7/1 -> Product2/2
  Trigonometric Function8/1 -> Product3/1
  Trigonometric Function9/1 -> Product3/2
  Product3/1 -> Gain1/1
  Product/1 -> Gain/1
  Trigonometric Function/1 -> A_01/1
  Gain/1 -> A_01/2
  Product1/1 -> A_01/3
  Trigonometric Function5/1 -> A_01/4
  Product2/1 -> A_01/5
  Gain1/1 -> A_01/6
  Constant/1 -> A_01/7
  Trigonometric Function10/1 -> A_01/8
  Trigonometric Function11/1 -> A_01/9
  a/1 -> Product5/1, Product4/1
  Trigonometric Function12/1 -> Product4/2
  Trigonometric Function13/1 -> Product5/2
  Product4/1 -> Vector Concatenate/1
  Product5/1 -> Vector Concatenate/2
  Constant5/1 -> Vector Concatenate/3
  A_01/1 -> Matrix Concatenation/1
  Vector Concatenate/1 -> Matrix Concatenation/2
  Matrix Concatenation/1 -> Matrix Concatenation1/1
  Constant1/1 -> Matrix Concatenation1/2
  Matrix Concatenation1/1 -> Out1/1, Display/1

== system Simulatore_COMAU_UBHAND_IV_manipolazione_interna/SCOPES/Direct_kinematic2/Direct_kinematic_hand/Direct_kinematic_thumb/Subsystem ==
  q : Inport
  alpha : Inport  Port=2
  a : Inport  Port=3
  A_01 : aerolibutil/Create 3x3 Matrix
  Constant : Constant  Value=0
  Constant1 : Constant  Value=[0 0 0 1]  VectorParams1D=off
  Constant5 : Constant  Value=d_f(1,1)
  Display : Display
  Gain : Gain  Gain=-1
  Gain1 : Gain  Gain=-1
  Matrix Concatenation : Concatenate  Mode=Multidimensional array  ConcatenateDimension=2
  Matrix Concatenation1 : Concatenate  Mode=Multidimensional array
  Product{,1..5} (6 alike) : Product  RndMeth=Zero
  Trigonometric Function{,1,6..8,11..12} (7 alike) : Trigonometry  Operator=cos
  Trigonometric Function{2..5,9..10,13} (7 alike) : Trigonometry
  Vector Concatenate : Concatenate  NumInputs=3  Mode=Multidimensional array
  Out1 : Outport
  wires:
  Trigonometric Function2/1 -> Product/1
  Trigonometric Function1/1 -> Product/2
  q/1 -> Trigonometric Function/1, Trigonometric Function2/1, Trigonometric Function3/1, Trigonometric Function5/1, Trigonometric Function6/1, Trigonometric Function8/1, Trigonometric Function12/1, Trigonometric Function13/1
  alpha/1 -> Trigonometric Function1/1, Trigonometric Function4/1, Trigonometric Function7/1, Trigonometric Function9/1, Trigonometric Function10/1, Trigonometric Function11/1
  Trigonometric Function3/1 -> Product1/1
  Trigonometric Function4/1 -> Product1/2
  Trigonometric Function6/1 -> Product2/1
  Trigonometric Function7/1 -> Product2/2
  Trigonometric Function8/1 -> Product3/1
  Trigonometric Function9/1 -> Product3/2
  Product3/1 -> Gain1/1
  Product/1 -> Gain/1
  Trigonometric Function/1 -> A_01/1
  Gain/1 -> A_01/2
  Product1/1 -> A_01/3
  Trigonometric Function5/1 -> A_01/4
  Product2/1 -> A_01/5
  Gain1/1 -> A_01/6
  Constant/1 -> A_01/7
  Trigonometric Function10/1 -> A_01/8
  Trigonometric Function11/1 -> A_01/9
  a/1 -> Product4/1, Product5/1
  Trigonometric Function12/1 -> Product4/2
  Trigonometric Function13/1 -> Product5/2
  Product4/1 -> Vector Concatenate/1
  Product5/1 -> Vector Concatenate/2
  Constant5/1 -> Vector Concatenate/3
  A_01/1 -> Matrix Concatenation/1
  Vector Concatenate/1 -> Matrix Concatenation/2
  Matrix Concatenation/1 -> Matrix Concatenation1/1
  Constant1/1 -> Matrix Concatenation1/2
  Matrix Concatenation1/1 -> Out1/1, Display/1

== system Simulatore_COMAU_UBHAND_IV_manipolazione_interna/SCOPES/Direct_kinematic2/Direct_kinematic_hand/Direct_kinematic_thumb/Subsystem1 ==
  q : Inport
  alpha : Inport  Port=2
  a : Inport  Port=3
  A_01 : aerolibutil/Create 3x3 Matrix
  Constant : Constant  Value=0
  Constant1 : Constant  Value=[0 0 0 1]  VectorParams1D=off
  Constant2 : Constant  Value=d_f(1,2)
  Display : Display
  Gain : Gain  Gain=-1
  Gain1 : Gain  Gain=-1
  Matrix Concatenation : Concatenate  Mode=Multidimensional array  ConcatenateDimension=2
  Matrix Concatenation1 : Concatenate  Mode=Multidimensional array
  Product{,1..5} (6 alike) : Product  RndMeth=Zero
  Trigonometric Function{,1,6..8,11..12} (7 alike) : Trigonometry  Operator=cos
  Trigonometric Function{2..5,9..10,13} (7 alike) : Trigonometry
  Vector Concatenate : Concatenate  NumInputs=3  Mode=Multidimensional array
  Out1 : Outport
  wires:
  Matrix Concatenation1/1 -> Display/1, Out1/1
  Constant1/1 -> Matrix Concatenation1/2
  Matrix Concatenation/1 -> Matrix Concatenation1/1
  Vector Concatenate/1 -> Matrix Concatenation/2
  A_01/1 -> Matrix Concatenation/1
  Constant2/1 -> Vector Concatenate/3
  Product5/1 -> Vector Concatenate/2
  Product4/1 -> Vector Concatenate/1
  Trigonometric Function13/1 -> Product5/2
  Trigonometric Function12/1 -> Product4/2
  a/1 -> Product4/1, Product5/1
  Trigonometric Function11/1 -> A_01/9
  Trigonometric Function10/1 -> A_01/8
  Constant/1 -> A_01/7
  Gain1/1 -> A_01/6
  Product2/1 -> A_01/5
  Trigonometric Function5/1 -> A_01/4
  Product1/1 -> A_01/3
  Gain/1 -> A_01/2
  Trigonometric Function/1 -> A_01/1
  Product/1 -> Gain/1
  Product3/1 -> Gain1/1
  Trigonometric Function9/1 -> Product3/2
  Trigonometric Function8/1 -> Product3/1
  Trigonometric Function7/1 -> Product2/2
  Trigonometric Function6/1 -> Product2/1
  Trigonometric Function4/1 -> Product1/2
  Trigonometric Function3/1 -> Product1/1
  alpha/1 -> Trigonometric Function11/1, Trigonometric Function10/1, Trigonometric Function9/1, Trigonometric Function7/1, Trigonometric Function4/1, Trigonometric Function1/1
  q/1 -> Trigonometric Function13/1, Trigonometric Function12/1, Trigonometric Function8/1, Trigonometric Function6/1, Trigonometric Function5/1, Trigonometric Function3/1, Trigonometric Function2/1, Trigonometric Function/1
  Trigonometric Function1/1 -> Product/2
  Trigonometric Function2/1 -> Product/1

== system Simulatore_COMAU_UBHAND_IV_manipolazione_interna/SCOPES/Direct_kinematic2/Direct_kinematic_hand/Direct_kinematic_thumb/Subsystem2 ==
  q : Inport
  alpha : Inport  Port=2
  a : Inport  Port=3
  A_01 : aerolibutil/Create 3x3 Matrix
  Constant : Constant  Value=0
  Constant1 : Constant  Value=[0 0 0 1]  VectorParams1D=off
  Constant5 : Constant  Value=d_f(1,3)
  Display : Display
  Gain : Gain  Gain=-1
  Gain1 : Gain  Gain=-1
  Matrix Concatenation : Concatenate  Mode=Multidimensional array  ConcatenateDimension=2
  Matrix Concatenation1 : Concatenate  Mode=Multidimensional array
  Product{,1..5} (6 alike) : Product  RndMeth=Zero
  Trigonometric Function{,1,6..8,11..12} (7 alike) : Trigonometry  Operator=cos
  Trigonometric Function{2..5,9..10,13} (7 alike) : Trigonometry
  Vector Concatenate : Concatenate  NumInputs=3  Mode=Multidimensional array
  Out1 : Outport
  wires:
  Trigonometric Function2/1 -> Product/1
  Trigonometric Function1/1 -> Product/2
  q/1 -> Trigonometric Function/1, Trigonometric Function2/1, Trigonometric Function3/1, Trigonometric Function5/1, Trigonometric Function6/1, Trigonometric Function8/1, Trigonometric Function12/1, Trigonometric Function13/1
  alpha/1 -> Trigonometric Function1/1, Trigonometric Function4/1, Trigonometric Function7/1, Trigonometric Function9/1, Trigonometric Function10/1, Trigonometric Function11/1
  Trigonometric Function3/1 -> Product1/1
  Trigonometric Function4/1 -> Product1/2
  Trigonometric Function6/1 -> Product2/1
  Trigonometric Function7/1 -> Product2/2
  Trigonometric Function8/1 -> Product3/1
  Trigonometric Function9/1 -> Product3/2
  Product3/1 -> Gain1/1
  Product/1 -> Gain/1
  Trigonometric Function/1 -> A_01/1
  Gain/1 -> A_01/2
  Product1/1 -> A_01/3
  Trigonometric Function5/1 -> A_01/4
  Product2/1 -> A_01/5
  Gain1/1 -> A_01/6
  Constant/1 -> A_01/7
  Trigonometric Function10/1 -> A_01/8
  Trigonometric Function11/1 -> A_01/9
  a/1 -> Product4/1, Product5/1
  Trigonometric Function12/1 -> Product4/2
  Trigonometric Function13/1 -> Product5/2
  Product4/1 -> Vector Concatenate/1
  Product5/1 -> Vector Concatenate/2
  Constant5/1 -> Vector Concatenate/3
  A_01/1 -> Matrix Concatenation/1
  Vector Concatenate/1 -> Matrix Concatenation/2
  Matrix Concatenation/1 -> Matrix Concatenation1/1
  Constant1/1 -> Matrix Concatenation1/2
  Matrix Concatenation1/1 -> Out1/1, Display/1

== system Simulatore_COMAU_UBHAND_IV_manipolazione_interna/SCOPES/Direct_kinematic2/Direct_kinematic_hand/Direct_kinematic_thumb/Subsystem3 ==
  q : Inport
  alpha : Inport  Port=2
  a : Inport  Port=3
  A_01 : aerolibutil/Create 3x3 Matrix
  Constant : Constant  Value=0
  Constant1 : Constant  Value=[0 0 0 1]  VectorParams1D=off
  Constant5 : Constant  Value=d_f(1,4)
  Display : Display
  Gain : Gain  Gain=-1
  Gain1 : Gain  Gain=-1
  Matrix Concatenation : Concatenate  Mode=Multidimensional array  ConcatenateDimension=2
  Matrix Concatenation1 : Concatenate  Mode=Multidimensional array
  Product{,1..5} (6 alike) : Product  RndMeth=Zero
  Trigonometric Function{,1,6..8,11..12} (7 alike) : Trigonometry  Operator=cos
  Trigonometric Function{2..5,9..10,13} (7 alike) : Trigonometry
  Vector Concatenate : Concatenate  NumInputs=3  Mode=Multidimensional array
  Out1 : Outport
  wires:
  Matrix Concatenation1/1 -> Display/1, Out1/1
  Constant1/1 -> Matrix Concatenation1/2
  Matrix Concatenation/1 -> Matrix Concatenation1/1
  Vector Concatenate/1 -> Matrix Concatenation/2
  A_01/1 -> Matrix Concatenation/1
  Constant5/1 -> Vector Concatenate/3
  Product5/1 -> Vector Concatenate/2
  Product4/1 -> Vector Concatenate/1
  Trigonometric Function13/1 -> Product5/2
  Trigonometric Function12/1 -> Product4/2
  a/1 -> Product4/1, Product5/1
  Trigonometric Function11/1 -> A_01/9
  Trigonometric Function10/1 -> A_01/8
  Constant/1 -> A_01/7
  Gain1/1 -> A_01/6
  Product2/1 -> A_01/5
  Trigonometric Function5/1 -> A_01/4
  Product1/1 -> A_01/3
  Gain/1 -> A_01/2
  Trigonometric Function/1 -> A_01/1
  Product/1 -> Gain/1
  Product3/1 -> Gain1/1
  Trigonometric Function9/1 -> Product3/2
  Trigonometric Function8/1 -> Product3/1
  Trigonometric Function7/1 -> Product2/2
  Trigonometric Function6/1 -> Product2/1
  Trigonometric Function4/1 -> Product1/2
  Trigonometric Function3/1 -> Product1/1
  alpha/1 -> Trigonometric Function11/1, Trigonometric Function10/1, Trigonometric Function9/1, Trigonometric Function7/1, Trigonometric Function4/1, Trigonometric Function1/1
  q/1 -> Trigonometric Function13/1, Trigonometric Function12/1, Trigonometric Function8/1, Trigonometric Function6/1, Trigonometric Function5/1, Trigonometric Function3/1, Trigonometric Function2/1, Trigonometric Function/1
  Trigonometric Function1/1 -> Product/2
  Trigonometric Function2/1 -> Product/1
